simulink model "MX_Gateway"  (Simulink 9.0)
settings: Solver=FixedStepDiscrete  StartTime=0.0  StopTime=100  FixedStep=0.01  RelTol=1e-3
values from neighbouring files (symbols this model uses):
  ACTIVE = Bobcat.Parameter; CreateDefine( ACTIVE                         ,1                          ,'Define' ,'defines.h' )    [defines.m]
  ACTIVE_MODE_ONE = Bobcat.Parameter; CreateDefine( ACTIVE_MODE_ONE                ,19                         ,'Define' ,'defines.h' )    [defines.m]
  AUTHENTICATED = Bobcat.Parameter; CreateDefine( AUTHENTICATED                  ,1                          ,'Define' ,'defines.h' )    [defines.m]
  AUX_EMEA_CONTROLS = Bobcat.Parameter; CreateDefine( AUX_EMEA_CONTROLS  ,1   ,'Define' ,'defines.h' )    [enums.m]
  AUX_FLOW_HIGH = Bobcat.Parameter; CreateDefine( AUX_FLOW_HIGH           ,4  ,'Define' ,'defines.h' )    [enums.m]
  AUX_FLOW_INACTIVE = Bobcat.Parameter; CreateDefine( AUX_FLOW_INACTIVE       ,0  ,'Define' ,'defines.h' )    [enums.m]
  AUX_FLOW_LOW = Bobcat.Parameter; CreateDefine( AUX_FLOW_LOW            ,1  ,'Define' ,'defines.h' )    [enums.m]
  AUX_FLOW_MEDIUM = Bobcat.Parameter; CreateDefine( AUX_FLOW_MEDIUM         ,2  ,'Define' ,'defines.h' )    [enums.m]
  AUX_FLOW_STANDARD = Bobcat.Parameter; CreateDefine( AUX_FLOW_STANDARD       ,3  ,'Define' ,'defines.h' )    [enums.m]
  AUX_NON_FUNCTIONAL = Bobcat.Parameter; CreateDefine( AUX_NON_FUNCTIONAL ,255 ,'Define' ,'defines.h' )    [enums.m]
  BASE_ACTIVE = Bobcat.Parameter; CreateDefine( BASE_ACTIVE                    ,253                        ,'Define' ,'defines.h' )    [defines.m]
  BASE_DETENT = Bobcat.Parameter; CreateDefine( BASE_DETENT                    ,1                          ,'Define' ,'defines.h' )    [defines.m]
  BEEP_NONE = Bobcat.Parameter; CreateDefine( BEEP_NONE                      ,255                        ,'Define' ,'defines.h' )    [defines.m]
  CD_AIR_FILTER_DEBOUNCE_NORMAL = Bobcat.Parameter; CreateAliasCD( CD_AIR_FILTER_DEBOUNCE_NORMAL                        ,uint16(500)                ,'ConstantDataPointer' ,'CONSTANT_DATA' ,'AIR_FILTER_DEBOUNCE_NORMAL')    [cdata.m]    (also in hc12_cdata.m; m4_cdata.m)
  CD_AIR_FILTER_DEBOUNCE_NOT_CONNECTED = Bobcat.Parameter; CreateAliasCD( CD_AIR_FILTER_DEBOUNCE_NOT_CONNECTED                 ,uint16(3000)               ,'ConstantDataPointer' ,'CONSTANT_DATA' ,'AIR_FILTER_DEBOUNCE_NOT_CONNECTED')    [cdata.m]    (also in hc12_cdata.m; m4_cdata.m)
  CD_AIR_FILTER_DEBOUNCE_PLUGGED = Bobcat.Parameter; CreateAliasCD( CD_AIR_FILTER_DEBOUNCE_PLUGGED                       ,uint16(3000)               ,'ConstantDataPointer' ,'CONSTANT_DATA' ,'AIR_FILTER_DEBOUNCE_PLUGGED')    [cdata.m]    (also in hc12_cdata.m; m4_cdata.m)
  CD_AIR_FILTER_FUNCTION = Bobcat.Parameter; CreateAliasCD( CD_AIR_FILTER_FUNCTION                               ,uint8(255)                 ,'ConstantDataPointer' ,'CONSTANT_DATA' ,'AIR_FILTER_FUNCTION')    [cdata.m]    (also in hc12_cdata.m; m4_cdata.m)
  CD_AIR_FILTER_PLUGGED_WEIGHT = Bobcat.Parameter; CreateAliasCD( CD_AIR_FILTER_PLUGGED_WEIGHT                         ,uint8(2)                   ,'ConstantDataPointer' ,'CONSTANT_DATA' ,'AIR_FILTER_PLUGGED_WEIGHT')    [cdata.m]    (also in hc12_cdata.m; m4_cdata.m)
  CD_AIR_FILTER_UNPLUGGED_WEIGHT = Bobcat.Parameter; CreateAliasCD( CD_AIR_FILTER_UNPLUGGED_WEIGHT                       ,uint8(1)                   ,'ConstantDataPointer' ,'CONSTANT_DATA' ,'AIR_FILTER_UNPLUGGED_WEIGHT')    [cdata.m]    (also in hc12_cdata.m; m4_cdata.m)
  CD_AUTOIDLE_ARMBAR_DELAY = Bobcat.Parameter; CreateAliasCD( CD_AUTOIDLE_ARMBAR_DELAY                             ,uint8(2)                   ,'ConstantDataPointer' ,'CONSTANT_DATA' ,'AUTOIDLE_ARMBAR_DELAY')    [cdata.m]    (also in hc12_cdata.m; m4_cdata.m)
  CD_AUTOIDLE_FUNCTION = Bobcat.Parameter; CreateAliasCD( CD_AUTOIDLE_FUNCTION                                 ,uint8(255)                 ,'ConstantDataPointer' ,'CONSTANT_DATA' ,'AUTOIDLE_FUNCTION')    [cdata.m]    (also in hc12_cdata.m; m4_cdata.m)
  CD_AUTOIDLE_IDLE_DELAY = Bobcat.Parameter; CreateAliasCD( CD_AUTOIDLE_IDLE_DELAY                               ,uint8(40)                  ,'ConstantDataPointer' ,'CONSTANT_DATA' ,'AUTOIDLE_IDLE_DELAY')    [cdata.m]    (also in hc12_cdata.m; m4_cdata.m)
  CD_AUTOIDLE_LOW_IDLE_START = Bobcat.Parameter; CreateAliasCD( CD_AUTOIDLE_LOW_IDLE_START                           ,uint8(255)                 ,'ConstantDataPointer' ,'CONSTANT_DATA' ,'AUTOIDLE_LOW_IDLE_START')    [cdata.m]    (also in hc12_cdata.m; m4_cdata.m)
  CD_AUTOIDLE_PRESSURE_OFF = Bobcat.Parameter; CreateAliasCD( CD_AUTOIDLE_PRESSURE_OFF                             ,uint16(300)                ,'ConstantDataPointer' ,'CONSTANT_DATA' ,'AUTOIDLE_PRESSURE_OFF')    [cdata.m]    (also in hc12_cdata.m; m4_cdata.m)
  CD_AUTOIDLE_PRESSURE_ON = Bobcat.Parameter; CreateAliasCD( CD_AUTOIDLE_PRESSURE_ON                              ,uint16(270)                ,'ConstantDataPointer' ,'CONSTANT_DATA' ,'AUTOIDLE_PRESSURE_ON')    [cdata.m]    (also in hc12_cdata.m; m4_cdata.m)
  CD_AUTOSHIFT_FUNCTION = Bobcat.Parameter; CreateAliasCD( CD_AUTOSHIFT_FUNCTION                                ,uint8(255)                 ,'ConstantDataPointer' ,'CONSTANT_DATA' ,'AUTOSHIFT_FUNCTION')    [cdata.m]    (also in hc12_cdata.m; m4_cdata.m)
  CD_AUTOSHIFT_PHIGH1 = Bobcat.Parameter; CreateAliasCD( CD_AUTOSHIFT_PHIGH1                                  ,uint16(205)                ,'ConstantDataPointer' ,'CONSTANT_DATA' ,'AUTOSHIFT_PHIGH1')    [cdata.m]    (also in hc12_cdata.m; m4_cdata.m)
  CD_AUTOSHIFT_PHIGH2 = Bobcat.Parameter; CreateAliasCD( CD_AUTOSHIFT_PHIGH2                                  ,uint16(193)                ,'ConstantDataPointer' ,'CONSTANT_DATA' ,'AUTOSHIFT_PHIGH2')    [cdata.m]    (also in hc12_cdata.m; m4_cdata.m)
  CD_AUTOSHIFT_PHIGH_TEMP1 = Bobcat.Parameter; CreateAliasCD( CD_AUTOSHIFT_PHIGH_TEMP1                             ,int16(70)                  ,'ConstantDataPointer' ,'CONSTANT_DATA' ,'AUTOSHIFT_PHIGH_TEMP1')    [cdata.m]    (also in hc12_cdata.m; m4_cdata.m)
  CD_AUTOSHIFT_PHIGH_TEMP2 = Bobcat.Parameter; CreateAliasCD( CD_AUTOSHIFT_PHIGH_TEMP2                             ,int16(160)                 ,'ConstantDataPointer' ,'CONSTANT_DATA' ,'AUTOSHIFT_PHIGH_TEMP2')    [cdata.m]    (also in hc12_cdata.m; m4_cdata.m)
  CD_AUTOSHIFT_PLOW1 = Bobcat.Parameter; CreateAliasCD( CD_AUTOSHIFT_PLOW1                                   ,uint16(130)                ,'ConstantDataPointer' ,'CONSTANT_DATA' ,'AUTOSHIFT_PLOW1')    [cdata.m]    (also in hc12_cdata.m; m4_cdata.m)
  CD_AUTOSHIFT_PLOW2 = Bobcat.Parameter; CreateAliasCD( CD_AUTOSHIFT_PLOW2                                   ,uint16(100)                ,'ConstantDataPointer' ,'CONSTANT_DATA' ,'AUTOSHIFT_PLOW2')    [cdata.m]    (also in hc12_cdata.m; m4_cdata.m)
  CD_AUTOSHIFT_PLOW_TEMP1 = Bobcat.Parameter; CreateAliasCD( CD_AUTOSHIFT_PLOW_TEMP1                              ,int16(20)                  ,'ConstantDataPointer' ,'CONSTANT_DATA' ,'AUTOSHIFT_PLOW_TEMP1')    [cdata.m]    (also in hc12_cdata.m; m4_cdata.m)
  CD_AUTOSHIFT_PLOW_TEMP2 = Bobcat.Parameter; CreateAliasCD( CD_AUTOSHIFT_PLOW_TEMP2                              ,int16(80)                  ,'ConstantDataPointer' ,'CONSTANT_DATA' ,'AUTOSHIFT_PLOW_TEMP2')    [cdata.m]    (also in hc12_cdata.m; m4_cdata.m)
  CD_AUTOSHIFT_TRAVEL_DELAY = Bobcat.Parameter; CreateAliasCD( CD_AUTOSHIFT_TRAVEL_DELAY                            ,uint8(32)                  ,'ConstantDataPointer' ,'CONSTANT_DATA' ,'AUTOSHIFT_TRAVEL_DELAY')    [cdata.m]    (also in hc12_cdata.m; m4_cdata.m)
  CD_AUX2_CRACKPOINT_CURRENT = Bobcat.Parameter; CreateAliasCD( CD_AUX2_CRACKPOINT_CURRENT                           ,fi(0,0,16,12,'hex','0c28') ,'ConstantDataPointer' ,'CONSTANT_DATA' ,'AUX2_CRACKPOINT_CURRENT')    [cdata.m]    (also in hc12_cdata.m; m4_cdata.m)
  CD_AUX2_FLOW_LOW_FUNCTION = Bobcat.Parameter; CreateAliasCD( CD_AUX2_FLOW_LOW_FUNCTION                            ,uint8(255)                 ,'ConstantDataPointer' ,'CONSTANT_DATA' ,'AUX2_FLOW_LOW_FUNCTION')    [cdata.m]    (also in hc12_cdata.m; m4_cdata.m)
  CD_AUX2_FLOW_MED_FUNCTION = Bobcat.Parameter; CreateAliasCD( CD_AUX2_FLOW_MED_FUNCTION                            ,uint8(255)                 ,'ConstantDataPointer' ,'CONSTANT_DATA' ,'AUX2_FLOW_MED_FUNCTION')    [cdata.m]    (also in hc12_cdata.m; m4_cdata.m)
  CD_AUX2_HI_FLOW_PERCENT = Bobcat.Parameter; CreateAliasCD( CD_AUX2_HI_FLOW_PERCENT                              ,fi(0,0,16,16,'hex','ffff') ,'ConstantDataPointer' ,'CONSTANT_DATA' ,'AUX2_HI_FLOW_PERCENT')    [cdata.m]    (also in hc12_cdata.m; m4_cdata.m)
  CD_AUX2_LOW_FLOW_PERCENT = Bobcat.Parameter; CreateAliasCD( CD_AUX2_LOW_FLOW_PERCENT                             ,fi(0,0,16,16,'hex','7333') ,'ConstantDataPointer' ,'CONSTANT_DATA' ,'AUX2_LOW_FLOW_PERCENT')    [cdata.m]    (also in hc12_cdata.m; m4_cdata.m)
  CD_AUX2_MAX_FLOW_CURRENT = Bobcat.Parameter; CreateAliasCD( CD_AUX2_MAX_FLOW_CURRENT                             ,fi(0,0,16,12,'hex','14cc') ,'ConstantDataPointer' ,'CONSTANT_DATA' ,'AUX2_MAX_FLOW_CURRENT')    [cdata.m]    (also in hc12_cdata.m; m4_cdata.m)
  CD_AUX2_MED_FLOW_PERCENT = Bobcat.Parameter; CreateAliasCD( CD_AUX2_MED_FLOW_PERCENT                             ,fi(0,0,16,16,'hex','9999') ,'ConstantDataPointer' ,'CONSTANT_DATA' ,'AUX2_MED_FLOW_PERCENT')    [cdata.m]    (also in hc12_cdata.m; m4_cdata.m)
  CD_AUX_CRACKPOINT_CURRENT = Bobcat.Parameter; CreateAliasCD( CD_AUX_CRACKPOINT_CURRENT                            ,fi(0,0,16,12,'hex','0000') ,'ConstantDataPointer' ,'CONSTANT_DATA' ,'AUX_CRACKPOINT_CURRENT')    [cdata.m]    (also in hc12_cdata.m; m4_cdata.m)
  CD_AUX_DETENT_FLASH_TIME = Bobcat.Parameter; CreateAliasCD( CD_AUX_DETENT_FLASH_TIME                             ,uint16(72)                 ,'ConstantDataPointer' ,'CONSTANT_DATA' ,'AUX_DETENT_FLASH_TIME')    [cdata.m]    (also in hc12_cdata.m; m4_cdata.m)
  CD_AUX_DETENT_HOLD_TIME = Bobcat.Parameter; CreateAliasCD( CD_AUX_DETENT_HOLD_TIME                              ,uint16(100)                ,'ConstantDataPointer' ,'CONSTANT_DATA' ,'AUX_DETENT_HOLD_TIME')    [cdata.m]    (also in hc12_cdata.m; m4_cdata.m)
  CD_AUX_FULL_ON_AUX1 = Bobcat.Parameter; CreateAliasCD( CD_AUX_FULL_ON_AUX1                                  ,fi(0,0,16,12,'hex','10cd') ,'ConstantDataPointer' ,'CONSTANT_DATA' ,'AUX_FULL_ON_AUX1')    [cdata.m]    (also in hc12_cdata.m; m4_cdata.m)
  CD_AUX_FULL_ON_AUX2 = Bobcat.Parameter; CreateAliasCD( CD_AUX_FULL_ON_AUX2                                  ,fi(0,0,16,12,'hex','139d') ,'ConstantDataPointer' ,'CONSTANT_DATA' ,'AUX_FULL_ON_AUX2')    [cdata.m]    (also in hc12_cdata.m; m4_cdata.m)
  CD_AUX_FULL_ON_AUX3 = Bobcat.Parameter; CreateAliasCD( CD_AUX_FULL_ON_AUX3                                  ,fi(0,0,16,12,'hex','17d7') ,'ConstantDataPointer' ,'CONSTANT_DATA' ,'AUX_FULL_ON_AUX3')    [cdata.m]    (also in hc12_cdata.m; m4_cdata.m)
  CD_AUX_FUNCTION = Bobcat.Parameter; CreateAliasCD( CD_AUX_FUNCTION                                      ,uint8(0)                   ,'ConstantDataPointer' ,'CONSTANT_DATA' ,'AUX_FUNCTION')    [cdata.m]    (also in hc12_cdata.m; m4_cdata.m)
  CD_AUX_RATE_LIMIT_CRACKPOINT = Bobcat.Parameter; CreateAliasCD( CD_AUX_RATE_LIMIT_CRACKPOINT                         ,fi(0,0,16,12,'hex','0800') ,'ConstantDataPointer' ,'CONSTANT_DATA' ,'AUX_RATE_LIMIT_CRACKPOINT')    [cdata.m]    (also in hc12_cdata.m; m4_cdata.m)
  CD_AUX_RATE_LIMIT_CRACKPOINT_RATE = Bobcat.Parameter; CreateAliasCD( CD_AUX_RATE_LIMIT_CRACKPOINT_RATE                    ,fi(0,1,32,12,'int',102400) ,'ConstantDataPointer' ,'CONSTANT_DATA' ,'AUX_RATE_LIMIT_CRACKPOINT_RATE')    [cdata.m]    (also in hc12_cdata.m; m4_cdata.m)
  CD_AUX_RATE_LIMIT_FUNCTION = Bobcat.Parameter; CreateAliasCD( CD_AUX_RATE_LIMIT_FUNCTION                           ,uint8(255)                 ,'ConstantDataPointer' ,'CONSTANT_DATA' ,'AUX_RATE_LIMIT_FUNCTION')    [cdata.m]    (also in hc12_cdata.m; m4_cdata.m)
  CD_AUX_RATE_LIMIT_MAX_ENG_SPD = Bobcat.Parameter; CreateAliasCD( CD_AUX_RATE_LIMIT_MAX_ENG_SPD                        ,uint16(1600)               ,'ConstantDataPointer' ,'CONSTANT_DATA' ,'AUX_RATE_LIMIT_MAX_ENG_SPD')    [cdata.m]    (also in hc12_cdata.m; m4_cdata.m)
  CD_AUX_RATE_LIMIT_MIN_ENG_SPD = Bobcat.Parameter; CreateAliasCD( CD_AUX_RATE_LIMIT_MIN_ENG_SPD                        ,uint16(100)                ,'ConstantDataPointer' ,'CONSTANT_DATA' ,'AUX_RATE_LIMIT_MIN_ENG_SPD')    [cdata.m]    (also in hc12_cdata.m; m4_cdata.m)
  CD_AUX_RATE_LIMIT_NORMAL_RATE = Bobcat.Parameter; CreateAliasCD( CD_AUX_RATE_LIMIT_NORMAL_RATE                        ,fi(0,1,32,12,'int',2048)   ,'ConstantDataPointer' ,'CONSTANT_DATA' ,'AUX_RATE_LIMIT_NORMAL_RATE')    [cdata.m]    (also in hc12_cdata.m; m4_cdata.m)
  CD_AUXPOT_CAL_DEADBAND_HIGH = Bobcat.Parameter; CreateAliasCD( CD_AUXPOT_CAL_DEADBAND_HIGH                          ,uint16(574)                ,'ConstantDataPointer' ,'CONSTANT_DATA' ,'AUXPOT_CAL_DEADBAND_HIGH')    [cdata.m]    (also in hc12_cdata.m; m4_cdata.m)
  CD_AUXPOT_CAL_DEADBAND_LOW = Bobcat.Parameter; CreateAliasCD( CD_AUXPOT_CAL_DEADBAND_LOW                           ,uint16(424)                ,'ConstantDataPointer' ,'CONSTANT_DATA' ,'AUXPOT_CAL_DEADBAND_LOW')    [cdata.m]    (also in hc12_cdata.m; m4_cdata.m)
  CD_AUXPOT_CENTER_RATIO = Bobcat.Parameter; CreateAliasCD( CD_AUXPOT_CENTER_RATIO                               ,uint16(500)                ,'ConstantDataPointer' ,'CONSTANT_DATA' ,'AUXPOT_CENTER_RATIO')    [cdata.m]    (also in hc12_cdata.m; m4_cdata.m)
  CD_AUXPOT_DEADBAND = Bobcat.Parameter; CreateAliasCD( CD_AUXPOT_DEADBAND                                   ,uint8(38)                  ,'ConstantDataPointer' ,'CONSTANT_DATA' ,'AUXPOT_DEADBAND')    [cdata.m]    (also in hc12_cdata.m; m4_cdata.m)
  CD_AUXPOT_FUNCTION = Bobcat.Parameter; CreateAliasCD( CD_AUXPOT_FUNCTION                                   ,uint8(0)                   ,'ConstantDataPointer' ,'CONSTANT_DATA' ,'AUXPOT_FUNCTION')    [cdata.m]    (also in hc12_cdata.m; m4_cdata.m)
  CD_AUXPOT_RATIO_GAIN = Bobcat.Parameter; CreateAliasCD( CD_AUXPOT_RATIO_GAIN                                 ,uint16(1000)               ,'ConstantDataPointer' ,'CONSTANT_DATA' ,'AUXPOT_RATIO_GAIN')    [cdata.m]    (also in hc12_cdata.m; m4_cdata.m)
  CD_CAN_THROTTLE_FUNCTION = Bobcat.Parameter; CreateAliasCD( CD_CAN_THROTTLE_FUNCTION                             ,uint8(255)                 ,'ConstantDataPointer' ,'CONSTANT_DATA' ,'CAN_THROTTLE_FUNCTION')    [cdata.m]    (also in hc12_cdata.m; m4_cdata.m)
  CD_CAN_THROTTLE_REVERSE = Bobcat.Parameter; CreateAliasCD( CD_CAN_THROTTLE_REVERSE                              ,uint8(255)                 ,'ConstantDataPointer' ,'CONSTANT_DATA' ,'CAN_THROTTLE_REVERSE')    [cdata.m]    (also in hc12_cdata.m; m4_cdata.m)
  CD_CONSOLE_DEBOUNCE_ABSENT = Bobcat.Parameter; CreateAliasCD( CD_CONSOLE_DEBOUNCE_ABSENT                           ,uint16(12)                 ,'ConstantDataPointer' ,'CONSTANT_DATA' ,'CONSOLE_DEBOUNCE_ABSENT')    [cdata.m]    (also in hc12_cdata.m; m4_cdata.m)
  CD_CONSOLE_DEBOUNCE_OORH = Bobcat.Parameter; CreateAliasCD( CD_CONSOLE_DEBOUNCE_OORH                             ,uint16(12)                 ,'ConstantDataPointer' ,'CONSTANT_DATA' ,'CONSOLE_DEBOUNCE_OORH')    [cdata.m]    (also in hc12_cdata.m; m4_cdata.m)
  CD_CONSOLE_DEBOUNCE_OORL = Bobcat.Parameter; CreateAliasCD( CD_CONSOLE_DEBOUNCE_OORL                             ,uint16(12)                 ,'ConstantDataPointer' ,'CONSTANT_DATA' ,'CONSOLE_DEBOUNCE_OORL')    [cdata.m]    (also in hc12_cdata.m; m4_cdata.m)
  CD_CONSOLE_DEBOUNCE_PRESENT = Bobcat.Parameter; CreateAliasCD( CD_CONSOLE_DEBOUNCE_PRESENT                          ,uint16(12)                 ,'ConstantDataPointer' ,'CONSTANT_DATA' ,'CONSOLE_DEBOUNCE_PRESENT')    [cdata.m]    (also in hc12_cdata.m; m4_cdata.m)
  CD_CONSOLE_FUNCTION = Bobcat.Parameter; CreateAliasCD( CD_CONSOLE_FUNCTION                                  ,uint8(0)                   ,'ConstantDataPointer' ,'CONSTANT_DATA' ,'CONSOLE_FUNCTION')    [cdata.m]    (also in hc12_cdata.m; m4_cdata.m)
  CD_CONSOLE_OORH = Bobcat.Parameter; CreateAliasCD( CD_CONSOLE_OORH                                      ,uint16(740)                ,'ConstantDataPointer' ,'CONSTANT_DATA' ,'CONSOLE_OORH')    [cdata.m]    (also in hc12_cdata.m; m4_cdata.m)
  CD_CONSOLE_OORL = Bobcat.Parameter; CreateAliasCD( CD_CONSOLE_OORL                                      ,uint16(10)                 ,'ConstantDataPointer' ,'CONSTANT_DATA' ,'CONSOLE_OORL')    [cdata.m]    (also in hc12_cdata.m; m4_cdata.m)
  CD_CONSOLE_PRESENT_HIGH = Bobcat.Parameter; CreateAliasCD( CD_CONSOLE_PRESENT_HIGH                              ,uint16(515)                ,'ConstantDataPointer' ,'CONSTANT_DATA' ,'CONSOLE_PRESENT_HIGH')    [cdata.m]    (also in hc12_cdata.m; m4_cdata.m)
  CD_CONSOLE_PRESENT_LOW = Bobcat.Parameter; CreateAliasCD( CD_CONSOLE_PRESENT_LOW                               ,uint16(375)                ,'ConstantDataPointer' ,'CONSTANT_DATA' ,'CONSOLE_PRESENT_LOW')    [cdata.m]    (also in hc12_cdata.m; m4_cdata.m)
  CD_DELUXE_GEN5_5_FUNCTION = Bobcat.Parameter; CreateAliasCD( CD_DELUXE_GEN5_5_FUNCTION                            ,uint8(255)                 ,'ConstantDataPointer' ,'CONSTANT_DATA' ,'DELUXE_GEN5_5_FUNCTION')    [cdata.m]    (also in hc12_cdata.m; m4_cdata.m)
  CD_DELUXE_GEN5_FUNCTION = Bobcat.Parameter; CreateAliasCD( CD_DELUXE_GEN5_FUNCTION                              ,uint8(255)                 ,'ConstantDataPointer' ,'CONSTANT_DATA' ,'DELUXE_GEN5_FUNCTION')    [cdata.m]    (also in hc12_cdata.m; m4_cdata.m)
  CD_DERATE_ENGINE_SPEED_DEBOUNCE_HIGH = Bobcat.Parameter; CreateAliasCD( CD_DERATE_ENGINE_SPEED_DEBOUNCE_HIGH                 ,uint16(200)                ,'ConstantDataPointer' ,'CONSTANT_DATA' ,'DERATE_ENGINE_SPEED_DEBOUNCE_HIGH')    [cdata.m]    (also in hc12_cdata.m; m4_cdata.m)
  CD_DERATE_ENGINE_SPEED_DEBOUNCE_SHUTDOWN = Bobcat.Parameter; CreateAliasCD( CD_DERATE_ENGINE_SPEED_DEBOUNCE_SHUTDOWN             ,uint16(1000)               ,'ConstantDataPointer' ,'CONSTANT_DATA' ,'DERATE_ENGINE_SPEED_DEBOUNCE_SHUTDOWN')    [cdata.m]    (also in hc12_cdata.m; m4_cdata.m)
  CD_DERATE_ENGINE_SPEED_HIGH_OFFSET = Bobcat.Parameter; CreateAliasCD( CD_DERATE_ENGINE_SPEED_HIGH_OFFSET                   ,uint16(200)                ,'ConstantDataPointer' ,'CONSTANT_DATA' ,'DERATE_ENGINE_SPEED_HIGH_OFFSET')    [cdata.m]    (also in hc12_cdata.m; m4_cdata.m)
  CD_DERATE_METHOD = Bobcat.Parameter; CreateAliasCD( CD_DERATE_METHOD                                     ,uint8(0)                   ,'ConstantDataPointer' ,'CONSTANT_DATA' ,'DERATE_METHOD')    [cdata.m]    (also in hc12_cdata.m; m4_cdata.m)
  CD_DUMP_VALVE_FUNCTION = Bobcat.Parameter; CreateAliasCD( CD_DUMP_VALVE_FUNCTION                               ,uint8(255)                 ,'ConstantDataPointer' ,'CONSTANT_DATA' ,'DUMP_VALVE_FUNCTION')    [cdata.m]    (also in hc12_cdata.m; m4_cdata.m)
  CD_DUMP_VALVE_ON_CURRENT = Bobcat.Parameter; CreateAliasCD( CD_DUMP_VALVE_ON_CURRENT                             ,fi(0,0,16,12,'hex','ffff') ,'ConstantDataPointer' ,'CONSTANT_DATA' ,'DUMP_VALVE_ON_CURRENT')    [cdata.m]    (also in hc12_cdata.m; m4_cdata.m)
  CD_ECO_MODE_THROTTLE_REDUCTION = Bobcat.Parameter; CreateAliasCD( CD_ECO_MODE_THROTTLE_REDUCTION                       ,uint16(850)                ,'ConstantDataPointer' ,'CONSTANT_DATA' ,'ECO_MODE_THROTTLE_REDUCTION')    [cdata.m]    (also in hc12_cdata.m; m4_cdata.m)
  CD_ECT_FILTER_GAIN = Bobcat.Parameter; CreateAliasCD( CD_ECT_FILTER_GAIN                                   ,uint8(6)                   ,'ConstantDataPointer' ,'CONSTANT_DATA' ,'ECT_FILTER_GAIN')    [cdata.m]    (also in hc12_cdata.m; m4_cdata.m)
  CD_ECU_FUNCTION = Bobcat.Parameter; CreateAliasCD( CD_ECU_FUNCTION                                      ,uint8(255)                 ,'ConstantDataPointer' ,'CONSTANT_DATA' ,'ECU_FUNCTION')    [cdata.m]    (also in hc12_cdata.m; m4_cdata.m)
  CD_ECU_HOLD_DEBOUNCE_ERROR_ON = Bobcat.Parameter; CreateAliasCD( CD_ECU_HOLD_DEBOUNCE_ERROR_ON                        ,uint16(20)                 ,'ConstantDataPointer' ,'CONSTANT_DATA' ,'ECU_HOLD_DEBOUNCE_ERROR_ON')    [cdata.m]    (also in hc12_cdata.m; m4_cdata.m)
  CD_EMEA_CONTROLS_HOLD_TIME = Bobcat.Parameter; CreateAliasCD( CD_EMEA_CONTROLS_HOLD_TIME                           ,uint16(30)                 ,'ConstantDataPointer' ,'CONSTANT_DATA' ,'EMEA_CONTROLS_HOLD_TIME')    [cdata.m]    (also in hc12_cdata.m; m4_cdata.m)
  CD_EMEA_CONTROLS_OPTION_A = Bobcat.Parameter; CreateAliasCD( CD_EMEA_CONTROLS_OPTION_A                            ,uint8(255)                 ,'ConstantDataPointer' ,'CONSTANT_DATA' ,'EMEA_CONTROLS_OPTION_A')    [cdata.m]    (also in hc12_cdata.m; m4_cdata.m)
  CD_EMEA_CONTROLS_OPTION_C = Bobcat.Parameter; CreateAliasCD( CD_EMEA_CONTROLS_OPTION_C                            ,uint8(255)                 ,'ConstantDataPointer' ,'CONSTANT_DATA' ,'EMEA_CONTROLS_OPTION_C')    [cdata.m]    (also in hc12_cdata.m; m4_cdata.m)
  CD_EMEA_CONTROLS_REVERSE = Bobcat.Parameter; CreateAliasCD( CD_EMEA_CONTROLS_REVERSE                             ,uint8(255)                 ,'ConstantDataPointer' ,'CONSTANT_DATA' ,'EMEA_CONTROLS_REVERSE')    [cdata.m]    (also in hc12_cdata.m; m4_cdata.m)
  CD_EMEA_ELECTRIC_VERSION = Bobcat.Parameter; CreateAliasCD( CD_EMEA_ELECTRIC_VERSION                             ,uint8(0)                   ,'ConstantDataPointer' ,'CONSTANT_DATA' ,'EMEA_ELECTRIC_VERSION')    [cdata.m]    (also in hc12_cdata.m; m4_cdata.m)
  CD_EMEA_POT_CAL_DEADBAND_HIGH = Bobcat.Parameter; CreateAliasCD( CD_EMEA_POT_CAL_DEADBAND_HIGH                        ,uint16(574)                ,'ConstantDataPointer' ,'CONSTANT_DATA' ,'EMEA_POT_CAL_DEADBAND_HIGH')    [cdata.m]    (also in hc12_cdata.m; m4_cdata.m)
  CD_EMEA_POT_CAL_DEADBAND_LOW = Bobcat.Parameter; CreateAliasCD( CD_EMEA_POT_CAL_DEADBAND_LOW                         ,uint16(424)                ,'ConstantDataPointer' ,'CONSTANT_DATA' ,'EMEA_POT_CAL_DEADBAND_LOW')    [cdata.m]    (also in hc12_cdata.m; m4_cdata.m)
  CD_ENGINE_RPM_FACTOR = Bobcat.Parameter; CreateAliasCD( CD_ENGINE_RPM_FACTOR                                 ,uint8(35)                  ,'ConstantDataPointer' ,'CONSTANT_DATA' ,'ENGINE_RPM_FACTOR')    [cdata.m]    (also in hc12_cdata.m; m4_cdata.m)
  CD_ENGINE_SPEED_FILTER_FAST_VALUE = Bobcat.Parameter; CreateAliasCD( CD_ENGINE_SPEED_FILTER_FAST_VALUE                    ,uint8(1)                   ,'ConstantDataPointer' ,'CONSTANT_DATA' ,'ENGINE_SPEED_FILTER_FAST_VALUE')    [cdata.m]    (also in hc12_cdata.m; m4_cdata.m)
  CD_ENGINE_SPEED_FILTER_SLOW_VALUE = Bobcat.Parameter; CreateAliasCD( CD_ENGINE_SPEED_FILTER_SLOW_VALUE                    ,uint8(4)                   ,'ConstantDataPointer' ,'CONSTANT_DATA' ,'ENGINE_SPEED_FILTER_SLOW_VALUE')    [cdata.m]    (also in hc12_cdata.m; m4_cdata.m)
  CD_ENGINE_SPEED_RUNNING = Bobcat.Parameter; CreateAliasCD( CD_ENGINE_SPEED_RUNNING                              ,uint16(800)                ,'ConstantDataPointer' ,'CONSTANT_DATA' ,'ENGINE_SPEED_RUNNING')    [cdata.m]    (also in hc12_cdata.m; m4_cdata.m)
  CD_ENGINE_START_DELAY_DEBOUNCE_RPM_NO_SIGNAL = Bobcat.Parameter; CreateAliasCD( CD_ENGINE_START_DELAY_DEBOUNCE_RPM_NO_SIGNAL         ,uint16(0)                  ,'ConstantDataPointer' ,'CONSTANT_DATA' ,'ENGINE_START_DELAY_DEBOUNCE_RPM_NO_SIGNAL')    [cdata.m]    (also in hc12_cdata.m; m4_cdata.m)
  CD_ENGINE_START_DELAY_OFF_TIME = Bobcat.Parameter; CreateAliasCD( CD_ENGINE_START_DELAY_OFF_TIME                       ,uint16(0)                  ,'ConstantDataPointer' ,'CONSTANT_DATA' ,'ENGINE_START_DELAY_OFF_TIME')    [cdata.m]    (also in hc12_cdata.m; m4_cdata.m)
  CD_ENGINE_START_DELAY_RPM_NO_SIGNAL = Bobcat.Parameter; CreateAliasCD( CD_ENGINE_START_DELAY_RPM_NO_SIGNAL                  ,uint16(0)                  ,'ConstantDataPointer' ,'CONSTANT_DATA' ,'ENGINE_START_DELAY_RPM_NO_SIGNAL')    [cdata.m]    (also in hc12_cdata.m; m4_cdata.m)
  CD_EOP_DEBOUNCE_EXTREMELY_LOW = Bobcat.Parameter; CreateAliasCD( CD_EOP_DEBOUNCE_EXTREMELY_LOW                        ,uint16(50)                 ,'ConstantDataPointer' ,'CONSTANT_DATA' ,'EOP_DEBOUNCE_EXTREMELY_LOW')    [cdata.m]    (also in hc12_cdata.m; m4_cdata.m)
  CD_EOP_DEBOUNCE_NORMAL = Bobcat.Parameter; CreateAliasCD( CD_EOP_DEBOUNCE_NORMAL                               ,uint16(50)                 ,'ConstantDataPointer' ,'CONSTANT_DATA' ,'EOP_DEBOUNCE_NORMAL')    [cdata.m]    (also in hc12_cdata.m; m4_cdata.m)
  CD_EOP_DEBOUNCE_SHUTDOWN = Bobcat.Parameter; CreateAliasCD( CD_EOP_DEBOUNCE_SHUTDOWN                             ,uint16(100)                ,'ConstantDataPointer' ,'CONSTANT_DATA' ,'EOP_DEBOUNCE_SHUTDOWN')    [cdata.m]    (also in hc12_cdata.m; m4_cdata.m)
  CD_EOP_FILTER_VALUE = Bobcat.Parameter; CreateAliasCD( CD_EOP_FILTER_VALUE                                  ,uint8(3)                   ,'ConstantDataPointer' ,'CONSTANT_DATA' ,'EOP_FILTER_VALUE')    [cdata.m]    (also in hc12_cdata.m; m4_cdata.m)
  CD_EOP_FUNCTION = Bobcat.Parameter; CreateAliasCD( CD_EOP_FUNCTION                                      ,uint8(1)                   ,'ConstantDataPointer' ,'CONSTANT_DATA' ,'EOP_FUNCTION')    [cdata.m]    (also in hc12_cdata.m; m4_cdata.m)
  CD_EOP_SWITCH_GAUGE_VALUE = Bobcat.Parameter; CreateAliasCD( CD_EOP_SWITCH_GAUGE_VALUE                            ,uint16(125)                ,'ConstantDataPointer' ,'CONSTANT_DATA' ,'EOP_SWITCH_GAUGE_VALUE')    [cdata.m]    (also in hc12_cdata.m; m4_cdata.m)
  CD_EOP_SWITCH_LOW = Bobcat.Parameter; CreateAliasCD( CD_EOP_SWITCH_LOW                                    ,uint16(50)                 ,'ConstantDataPointer' ,'CONSTANT_DATA' ,'EOP_SWITCH_LOW')    [cdata.m]    (also in hc12_cdata.m; m4_cdata.m)
  CD_EOP_SWITCH_PRESSURE_VALUE = Bobcat.Parameter; CreateAliasCD( CD_EOP_SWITCH_PRESSURE_VALUE                         ,uint16(40)                 ,'ConstantDataPointer' ,'CONSTANT_DATA' ,'EOP_SWITCH_PRESSURE_VALUE')    [cdata.m]    (also in hc12_cdata.m; m4_cdata.m)
  CD_EOT_DEBOUNCE_EXTREMELY_LOW = Bobcat.Parameter; CreateAliasCD( CD_EOT_DEBOUNCE_EXTREMELY_LOW                        ,uint16(2)                  ,'ConstantDataPointer' ,'CONSTANT_DATA' ,'EOT_DEBOUNCE_EXTREMELY_LOW')    [cdata.m]    (also in hc12_cdata.m; m4_cdata.m)
  CD_EOT_DEBOUNCE_LOW = Bobcat.Parameter; CreateAliasCD( CD_EOT_DEBOUNCE_LOW                                  ,uint16(2)                  ,'ConstantDataPointer' ,'CONSTANT_DATA' ,'EOT_DEBOUNCE_LOW')    [cdata.m]    (also in hc12_cdata.m; m4_cdata.m)
  CD_EOT_DEBOUNCE_NORMAL = Bobcat.Parameter; CreateAliasCD( CD_EOT_DEBOUNCE_NORMAL                               ,uint16(2)                  ,'ConstantDataPointer' ,'CONSTANT_DATA' ,'EOT_DEBOUNCE_NORMAL')    [cdata.m]    (also in hc12_cdata.m; m4_cdata.m)
  CD_EOT_EXTREMELY_LOW = Bobcat.Parameter; CreateAliasCD( CD_EOT_EXTREMELY_LOW                                 ,uint16(81)                 ,'ConstantDataPointer' ,'CONSTANT_DATA' ,'EOT_EXTREMELY_LOW')    [cdata.m]    (also in hc12_cdata.m; m4_cdata.m)
  CD_EOT_FUNCTION = Bobcat.Parameter; CreateAliasCD( CD_EOT_FUNCTION                                      ,uint8(255)                 ,'ConstantDataPointer' ,'CONSTANT_DATA' ,'EOT_FUNCTION')    [cdata.m]    (also in hc12_cdata.m; m4_cdata.m)
  CD_EOT_LOW = Bobcat.Parameter; CreateAliasCD( CD_EOT_LOW                                           ,uint16(90)                 ,'ConstantDataPointer' ,'CONSTANT_DATA' ,'EOT_LOW')    [cdata.m]    (also in hc12_cdata.m; m4_cdata.m)
  CD_EOT_TEMPERATURE_HYSTERESIS = Bobcat.Parameter; CreateAliasCD( CD_EOT_TEMPERATURE_HYSTERESIS                        ,uint16(2)                  ,'ConstantDataPointer' ,'CONSTANT_DATA' ,'EOT_TEMPERATURE_HYSTERESIS')    [cdata.m]    (also in hc12_cdata.m; m4_cdata.m)
  CD_FAST_TRAVEL_FUNCTION = Bobcat.Parameter; CreateAliasCD( CD_FAST_TRAVEL_FUNCTION                              ,uint8(255)                 ,'ConstantDataPointer' ,'CONSTANT_DATA' ,'FAST_TRAVEL_FUNCTION')    [cdata.m]    (also in hc12_cdata.m; m4_cdata.m)
  CD_FAST_TRAVEL_THROTTLE_DELAY = Bobcat.Parameter; CreateAliasCD( CD_FAST_TRAVEL_THROTTLE_DELAY                        ,uint16(10)                 ,'ConstantDataPointer' ,'CONSTANT_DATA' ,'FAST_TRAVEL_THROTTLE_DELAY')    [cdata.m]    (also in hc12_cdata.m; m4_cdata.m)
  CD_FAST_TRAVEL_THROTTLE_LIMIT = Bobcat.Parameter; CreateAliasCD( CD_FAST_TRAVEL_THROTTLE_LIMIT                        ,uint16(1000)               ,'ConstantDataPointer' ,'CONSTANT_DATA' ,'FAST_TRAVEL_THROTTLE_LIMIT')    [cdata.m]    (also in hc12_cdata.m; m4_cdata.m)
  CD_FLYWHEEL_TEETH = Bobcat.Parameter; CreateAliasCD( CD_FLYWHEEL_TEETH                                    ,uint8(83)                  ,'ConstantDataPointer' ,'CONSTANT_DATA' ,'FLYWHEEL_TEETH')    [cdata.m]    (also in hc12_cdata.m; m4_cdata.m)
  CD_FUEL_LEVEL_FILTER_FAST_VALUE = Bobcat.Parameter; CreateAliasCD( CD_FUEL_LEVEL_FILTER_FAST_VALUE                      ,uint8(5)                   ,'ConstantDataPointer' ,'CONSTANT_DATA' ,'FUEL_LEVEL_FILTER_FAST_VALUE')    [cdata.m]    (also in hc12_cdata.m; m4_cdata.m)
  CD_FUEL_LEVEL_FILTER_SLOW_VALUE = Bobcat.Parameter; CreateAliasCD( CD_FUEL_LEVEL_FILTER_SLOW_VALUE                      ,uint8(10)                  ,'ConstantDataPointer' ,'CONSTANT_DATA' ,'FUEL_LEVEL_FILTER_SLOW_VALUE')    [cdata.m]    (also in hc12_cdata.m; m4_cdata.m)
  CD_FUEL_PRIMARY_FEEDBACK_FUNCTION = Bobcat.Parameter; CreateAliasCD( CD_FUEL_PRIMARY_FEEDBACK_FUNCTION                    ,uint8(0)                   ,'ConstantDataPointer' ,'CONSTANT_DATA' ,'FUEL_PRIMARY_FEEDBACK_FUNCTION')    [cdata.m]    (also in hc12_cdata.m; m4_cdata.m)
  ... (+246 more)
undefined symbols (not defined in the file or in its folder): sigbuilder_block
undefined symbols (not defined in the file): CD_FUEL_RESOLUTION_TIMER
libraries: simulink x186, MX_Library x107
(part 43 of 48)

== stateflow chart MX_Gtwy_Control/PanelInterface/Keyless_Indicators/Keyless_Ind ==
  settings: executeAtInitialization=1
  state LOCK [parallel]
    settings: executionOrder=1
    state ACTIVE
      en:ind_Lock = ACTIVE;
    state INACTIVE
      en:ind_Lock = INACTIVE;
    state FAIL
      state ACTIVE
        en:ind_Lock = ACTIVE;
      state INACTIVE
        en:ind_Lock = INACTIVE;
      (default) -> LOCK.FAIL.ACTIVE
      LOCK.FAIL.INACTIVE -> LOCK.FAIL.ACTIVE : after(CD_ICON_FLASH_RATE, tick)
      LOCK.FAIL.ACTIVE -> LOCK.FAIL.INACTIVE : after(CD_ICON_FLASH_RATE,tick)
    (default) -> LOCK.INACTIVE
    LOCK.INACTIVE -> LOCK.ACTIVE [order 1] : [(ee_PasswordLock == ACTIVE)]
    LOCK.ACTIVE -> LOCK.INACTIVE [order 2] : [(ee_PasswordLock == INACTIVE)]
    LOCK.FAIL -> LOCK.ACTIVE [order 1] : [(state_KSPassword != FAIL) &&...
        |  (state_KSPassword != ENTER_PWD) &&...
        |  (ee_PasswordLock == ACTIVE) &&...
        |  (state_KSLock != KS_LOCK_MACHINE) &&...
        |  (state_KSLock != KS_CHANGE)]
    LOCK.ACTIVE -> LOCK.FAIL [order 1] : [(state_KSPassword == FAIL) ||...
        |  ((state_KSPassword == ENTER_PWD) &&...
        |  (state_KSLock != KS_UNLOCK_MACHINE)) ||...
        |  (state_KSLock == KS_CHANGE)]
    LOCK.INACTIVE -> LOCK.FAIL [order 2] : [(state_KSPassword == FAIL)...
        | || (state_KSLock == KS_LOCK_MACHINE)...
        | || (state_KSLock == KS_CHANGE)]
    LOCK.FAIL -> LOCK.INACTIVE [order 2] : [(state_KSPassword != FAIL) &&...
        |  (state_KSPassword != ENTER_PWD) &&...
        |  (ee_PasswordLock == INACTIVE) &&...
        |  (state_KSLock != KS_LOCK_MACHINE) &&...
        |  (state_KSLock != KS_CHANGE)]
  state UNLOCK [parallel]
    settings: executionOrder=2
    state INACTIVE
      en:ind_Unlock = INACTIVE;
    state ACTIVE
      en:ind_Unlock = ACTIVE;
    state FAIL
      state INACTIVE
        en:ind_Unlock = INACTIVE;
      state ACTIVE
        en:ind_Unlock = ACTIVE;
      (default) -> UNLOCK.FAIL.ACTIVE
      UNLOCK.FAIL.ACTIVE -> UNLOCK.FAIL.INACTIVE : after(CD_ICON_FLASH_RATE,tick)
      UNLOCK.FAIL.INACTIVE -> UNLOCK.FAIL.ACTIVE : after(CD_ICON_FLASH_RATE, tick)
    (default) -> UNLOCK.INACTIVE
    UNLOCK.INACTIVE -> UNLOCK.ACTIVE [order 1] : [(ee_PasswordLock == INACTIVE)]
    UNLOCK.ACTIVE -> UNLOCK.INACTIVE [order 2] : [(ee_PasswordLock == ACTIVE)]
    UNLOCK.FAIL -> UNLOCK.INACTIVE [order 1] : [(state_KSPassword != FAIL) &&...
        |  (ee_PasswordLock == ACTIVE) &&...
        |  (state_KSLock != KS_UNLOCK_MACHINE) &&...
        |  (state_KSLock != KS_CHANGE)]
    UNLOCK.INACTIVE -> UNLOCK.FAIL [order 2] : [(state_KSPassword == FAIL) ||...
        |  (state_KSLock == KS_UNLOCK_MACHINE) ||...
        |  (state_KSLock == KS_CHANGE)]
    UNLOCK.FAIL -> UNLOCK.ACTIVE [order 2] : [(state_KSPassword != FAIL) &&...
        |  (ee_PasswordLock == INACTIVE) &&...
        |  (state_KSLock != KS_UNLOCK_MACHINE) &&...
        |  (state_KSLock != KS_CHANGE)]
    UNLOCK.ACTIVE -> UNLOCK.FAIL [order 1] : [(state_KSPassword == FAIL) ||...
        |  (state_KSLock == KS_CHANGE)]
  data: ind_Lock output boolean; ACTIVE parameter uint8; INACTIVE parameter uint8; ind_Unlock output boolean; FAIL parameter uint8; state_KSPassword input uint8; CD_ICON_FLASH_RATE parameter uint16; state_KSLock input uint8; KS_LOCK_MACHINE parameter uint8; KS_CHANGE parameter uint8; KS_UNLOCK_MACHINE parameter uint8; ee_PasswordLock data_store_memory double; ENTER_PWD parameter uint8

== stateflow chart MX_Gtwy_Control/Inputs/Eco_Mode/Eco_Mode ==
  state Normal
    state On
      en:
      state_EcoMode = ACTIVE;
      ee_EcoMode = ACTIVE;
      matlab_UpdateEEVar();
    state Init
      en:
      state_EcoMode = INACTIVE;
    state Off
      en:
      state_EcoMode = INACTIVE;
      ee_EcoMode = INACTIVE;
      matlab_UpdateEEVar();
    (default) -> Init
    Init -> (junction 5179) : [flag_InitEERead]
    (junction 5179) -> Normal.On [order 1] : [ee_EcoMode == ACTIVE &&...
        | (ee_DeluxeG5 == ACTIVE ||...
        |  CD_DELUXE_GEN5_5_FUNCTION != NON_FUNCTIONAL)]
    (junction 5179) -> (junction 5180) [order 2]
    Normal.On -> Normal.Off : [button_EcoMode]
    Normal.Off -> Normal.On : [button_EcoMode]
    (junction 5180) -> Normal.Off
  state Deluxe_G55
    state Off
      en:
      state_EcoMode = INACTIVE;
    state On
      en:
      state_EcoMode = ACTIVE;
    (default) -> Deluxe_G55.Off
    Deluxe_G55.Off -> Deluxe_G55.On : [dlx_G55_EcoMode == ACTIVE]
    Deluxe_G55.On -> Deluxe_G55.Off : [dlx_G55_EcoMode != ACTIVE]
  (default) -> (junction 5201)
  (junction 5201) -> Normal [order 1] : [CD_DELUXE_GEN5_5_FUNCTION == NON_FUNCTIONAL]
  (junction 5201) -> Deluxe_G55 [order 2]
  data: state_EcoMode output uint8; button_EcoMode input boolean; INACTIVE parameter uint8; ACTIVE parameter uint8; ee_EcoMode data_store_memory double; ee_DeluxeG5 data_store_memory double; flag_InitEERead input boolean; CD_DELUXE_GEN5_5_FUNCTION parameter uint8; NON_FUNCTIONAL parameter uint8; dlx_G55_EcoMode input uint8

== stateflow chart MX_Gtwy_Control/PanelInterface/mod_BatteryIcon/BatteryIcon ==
  settings: executeAtInitialization=1
  state ERROR
    en:icon_Battery = ERROR_FLASH;
  state INACTIVE
    en:icon_Battery = INACTIVE;
  state ACTIVE
    en:icon_Battery = ACTIVE;
  (default) -> INACTIVE
  INACTIVE -> ERROR [order 1] : [(state_Alternator != NORMAL)...
      | || (state_VBattery != NORMAL)]
  ERROR -> INACTIVE : [(state_Alternator == NORMAL)...
      | && (state_VBattery == NORMAL)]
  INACTIVE -> ACTIVE [order 2] : [(state_InfoPrimary == DISPLAY_BATTERY_VOLTAGE)]
  ACTIVE -> INACTIVE [order 1] : [(state_InfoPrimary != DISPLAY_BATTERY_VOLTAGE)]
  ACTIVE -> ERROR [order 2] : [(state_Alternator != NORMAL)...
      | || (state_VBattery != NORMAL)]
  data: state_VBattery input uint8; icon_Battery output uint8; ACTIVE parameter uint8; INACTIVE parameter uint8; NORMAL parameter uint8; state_InfoPrimary input uint8; DISPLAY_BATTERY_VOLTAGE parameter uint8; state_Alternator input uint8; ERROR_FLASH parameter uint8

== stateflow chart MX_Gtwy_Control/PanelInterface/mod_ECTIcon/WarningIcon ==
  settings: executeAtInitialization=1
  state INACTIVE
    en:icon_ECT = INACTIVE;
  state ERROR
    en:icon_ECT = ERROR_FLASH;
  state ACTIVE
    en:icon_ECT = ACTIVE;
  (default) -> INACTIVE
  INACTIVE -> ERROR [order 1] : [(state_ECT == EXTREMELY_HIGH)...
      | || (state_ECT == PRE_SHUTDOWN)...
      | || (state_ECT == SHUTDOWN)]
  ERROR -> INACTIVE : [(state_ECT == HIGH)...
      | || (state_ECT == NORMAL)...
      | || (state_ECT == COLD)]
  ACTIVE -> INACTIVE [order 1] : [(state_ECT == NORMAL)...
      | || (state_ECT == COLD)]
  INACTIVE -> ACTIVE [order 2] : [(state_ECT == HIGH)...
      | || (state_ECT == OORH)...
      | || (state_ECT == OORL)]
  ACTIVE -> ERROR [order 2] : [(state_ECT == EXTREMELY_HIGH)...
      | || (state_ECT == PRE_SHUTDOWN)...
      | || (state_ECT == SHUTDOWN)]
  data: state_ECT input uint8; icon_ECT output uint8; ACTIVE parameter uint8; EXTREMELY_HIGH parameter uint8; INACTIVE parameter uint8; NORMAL parameter uint8; OORH parameter uint8; OORL parameter uint8; SHUTDOWN parameter uint8; HIGH parameter uint8; COLD parameter uint8; ERROR_FLASH parameter uint8; PRE_SHUTDOWN parameter uint8

== stateflow chart MX_Gtwy_Control/PanelInterface/mod_EngineIcon/EngineIcon ==
  settings: executeAtInitialization=1
  state SOLID
    state INACTIVE
      en:icon_Engine = INACTIVE;
    state ACTIVE
      en:icon_Engine = ACTIVE;
    (default) -> INACTIVE
    INACTIVE -> ACTIVE : [(state_StarterOutput != NORMAL)...
        | || (state_StarterRelay != NORMAL)...
        | || (state_EOP == LOW)...
        | || (state_EOP == OORL)...
        | || (state_EOP == OORH)...
        | || ((state_ThrottlePrimary != NORMAL) && (state_ThrottlePrimary != NON_FUNCTIONAL))...
        | || ((lamp_Engine & PROTECT_LAMP_STATUS_MASK) == PROTECT_LAMP_STATUS_ACTIVE)...
        | || ((lamp_Engine & AMBER_LAMP_STATUS_MASK) == AMBER_LAMP_STATUS_ACTIVE)...
        | || (state_EngineSpeedDerate == UNRESPONSIVE)...
        | || ((state_FuelTemp != NORMAL) && (state_FuelTemp != NON_FUNCTIONAL))...
        | || (icon_RPM == ERROR_FLASH && ee_DeluxeG5 == ACTIVE)...
        | || (state_GlowPlugOutput != NORMAL && ee_DeluxeG5 == ACTIVE)...
        | || (state_GlowPlugRelay != NORMAL && ee_DeluxeG5 == ACTIVE)...
        | || (state_TurboPrime != NORMAL)]
    ACTIVE -> INACTIVE : [(state_StarterOutput == NORMAL)...
        | && (state_StarterRelay == NORMAL)...
        | && (state_EOP == NORMAL)...
        | && ((state_ThrottlePrimary == NORMAL) || (state_ThrottlePrimary == NON_FUNCTIONAL))...
        | && ((lamp_Engine & PROTECT_LAMP_STATUS_MASK) != PROTECT_LAMP_STATUS_ACTIVE)...
        | && ((lamp_Engine & AMBER_LAMP_STATUS_MASK) != AMBER_LAMP_STATUS_ACTIVE)...
        | && (state_EngineSpeedDerate != UNRESPONSIVE)...
        | && ((state_FuelTemp == NORMAL) || (state_FuelTemp == NON_FUNCTIONAL))...
        | && (icon_RPM != ERROR_FLASH || ee_DeluxeG5 != ACTIVE)...
        | && (state_GlowPlugOutput == NORMAL || ee_DeluxeG5 != ACTIVE)...
        | && (state_GlowPlugRelay == NORMAL || ee_DeluxeG5 != ACTIVE)...
        | && (state_TurboPrime == NORMAL)]
  state ERROR
    en:icon_Engine = ERROR_FLASH;
  (default) -> SOLID
  SOLID -> ERROR : [(state_EOP == EXTREMELY_LOW)...
      | || (state_EOP == SHUTDOWN)...
      | || (error_FuelSec != NORMAL)...
      | || (state_FuelPriOutput != NORMAL)...
      | || (state_FuelPriRelay != NORMAL)...
      | || (state_HPMatch == FAILURE)...
      | || (state_CommECU == NO_COMMUNICATION)...
      | || ((lamp_Engine & RED_STOP_LAMP_STATUS_MASK) == RED_STOP_LAMP_STATUS_ACTIVE)...
      | || ((lamp_Engine & PROTECT_LAMP_FLASH_MASK) ==PROTECT_LAMP_FLASH_ACTIVE)...
      | || (state_EngineSpeedDerate == SHUTDOWN)...
      | || (state_FuelTemp == SHUTDOWN)]
  ERROR -> SOLID : [((state_EOP == NORMAL) || (state_EOP == LOW))...
      | && (error_FuelSec == NORMAL)...
      | && (state_FuelPriOutput == NORMAL)...
      | && (state_FuelPriRelay == NORMAL)...
      | && (state_HPMatch != FAILURE)...
      | && (state_CommECU != NO_COMMUNICATION)...
      | && ((lamp_Engine & RED_STOP_LAMP_STATUS_MASK) != RED_STOP_LAMP_STATUS_ACTIVE)...
      | && ((lamp_Engine & PROTECT_LAMP_FLASH_MASK) != PROTECT_LAMP_FLASH_ACTIVE)...
      | && (state_EngineSpeedDerate != SHUTDOWN)...
      | && (state_FuelTemp != SHUTDOWN)]
  data: icon_Engine output uint8; state_FuelPriOutput input uint8 [0..255]; state_FuelPriRelay input uint8 [0..255]; error_FuelSec input uint8 [0..255]; state_StarterOutput input uint8; state_StarterRelay input uint8; state_EOP input uint8 [0..255]; ACTIVE parameter uint8; EXTREMELY_LOW parameter uint8; INACTIVE parameter uint8; LOW parameter uint8; NORMAL parameter uint8; SHUTDOWN parameter uint8; OORL parameter uint8; OORH parameter uint8; ERROR_FLASH parameter uint8; state_ThrottlePrimary input uint8; state_CommECU input uint8; NO_COMMUNICATION parameter uint8; lamp_Engine input uint16; PROTECT_LAMP_STATUS_MASK parameter uint16; PROTECT_LAMP_STATUS_ACTIVE parameter uint16; AMBER_LAMP_STATUS_ACTIVE parameter uint16; RED_STOP_LAMP_STATUS_MASK parameter uint16; RED_STOP_LAMP_STATUS_ACTIVE parameter uint16; AMBER_LAMP_STATUS_MASK parameter uint16; NON_FUNCTIONAL parameter uint8; UNRESPONSIVE parameter uint8; state_EngineSpeedDerate input uint8; state_HPMatch input uint8 [0..255]; icon_RPM input uint8; ee_DeluxeG5 data_store_memory double; state_GlowPlugOutput input uint8; state_GlowPlugRelay input uint8; FAILURE parameter uint8; state_FuelTemp input uint8; PROTECT_LAMP_FLASH_ACTIVE parameter uint16; PROTECT_LAMP_FLASH_MASK parameter uint16; state_TurboPrime input uint8

== stateflow chart MX_Gtwy_Control/PanelInterface/mod_FuelLevelIcon/FuelLevel ==
  settings: executeAtInitialization=1
  state INACTIVE
    en:icon_Fuel = INACTIVE;
  state ACTIVE
    en:icon_Fuel = ACTIVE;
  (default) -> INACTIVE
  INACTIVE -> ACTIVE : [(state_FuelLevel != NORMAL)]
  ACTIVE -> INACTIVE : [(state_FuelLevel == NORMAL)]
  data: state_FuelLevel input uint8; icon_Fuel output boolean; ACTIVE parameter uint8; INACTIVE parameter uint8; NORMAL parameter uint8

== stateflow chart MX_Gtwy_Control/PanelInterface/mod_GeneralWarningIcon/WarningIcon ==
  settings: executeAtInitialization=1
  state SOLID
    state INACTIVE
      du:icon_General = INACTIVE;
    state ACTIVE
      en:icon_General = ACTIVE;
    (default) -> INACTIVE
    INACTIVE -> ACTIVE : [(state_SwPwrOutput != NORMAL)...
        | || (state_SwPwrRelay != NORMAL)...
        | || (state_PBValveOutput != NORMAL)...
        | || (state_PBValveRelay != NORMAL)...
        | || (state_SecondAux != ACTIVE && state_SecondAux != INACTIVE)...
        | || (state_SensorSupply1 != NORMAL)...
        | || (state_SensorSupply2 != NORMAL)...
        | || (state_ConstantData != NORMAL)...
        | || (state_DeluxeG5 == NO_COMMUNICATION)...
        | || (state_HVACOutput != NORMAL)...
        | || (state_OffsetBase != ACTIVE && state_OffsetBase != INACTIVE)...
        | || (state_OffsetRod != ACTIVE && state_OffsetRod != INACTIVE )...
        | || (state_OffsetPot == OORL)...
        | || (state_OffsetPot == OORH)...
        | || (state_OffsetPot == NOT_IN_NEUTRAL)...
        | || (state_AuxBase != ACTIVE && state_AuxBase != INACTIVE)...
        | || (state_AuxRod != ACTIVE && state_AuxRod != INACTIVE )...
        | || (state_AuxPot == OORL)...
        | || (state_AuxPot == OORH)...
        | || (state_AuxPot == NOT_IN_NEUTRAL)...
        | || (state_LeftPot == OORL)...
        | || (state_LeftPot == OORH)...
        | || (state_LeftPot == NOT_IN_NEUTRAL)...
        | || (state_RightPot == OORL)...
        | || (state_RightPot == OORH)...
        | || (state_RightPot == NOT_IN_NEUTRAL)...
        | || (state_LoadSense != NORMAL)...
        | || (state_HydExchange != NORMAL)...
        | || (state_CommThrottle == NO_COMMUNICATION)...
        | || (state_CommSecondary == NO_COMMUNICATION)...
        | || (state_CommJogShuttle == NO_COMMUNICATION)...
        | || (state_CommStdPanel == NO_COMMUNICATION)...
        | || (state_CommDeluxeG55 == NO_COMMUNICATION)...
        | || (state_CANThrottle  != NORMAL)...
        | || (ee_ThrottleCalState != NORMAL && CD_CAN_THROTTLE_FUNCTION != NON_FUNCTIONAL)...
        | || (icon_Service == ERROR_FLASH && ee_DeluxeG5 == ACTIVE) ]
    ACTIVE -> INACTIVE : [(state_SwPwrOutput == NORMAL)...
        | && (state_SwPwrRelay == NORMAL)...
        | && (state_PBValveOutput == NORMAL)...
        | && (state_PBValveRelay == NORMAL)...
        | && (state_SecondAux == ACTIVE || state_SecondAux == INACTIVE)...
        | && (state_SensorSupply1 == NORMAL)...
        | && (state_SensorSupply2 == NORMAL)...
        | && (state_DeluxeG5 != NO_COMMUNICATION)...
        | && (state_ConstantData == NORMAL)...
        | && (state_HVACOutput == NORMAL)...
        | && (state_OffsetBase == ACTIVE || state_OffsetBase == INACTIVE)...
        | && (state_OffsetRod == ACTIVE || state_OffsetRod == INACTIVE)...
        | && (state_OffsetPot != OORL)...
        | && (state_OffsetPot != OORH)...
        | && (state_OffsetPot != NOT_IN_NEUTRAL)...
        | && (state_AuxBase == ACTIVE || state_AuxBase == INACTIVE)...
        | && (state_AuxRod == ACTIVE || state_AuxRod == INACTIVE)...
        | && (state_AuxPot != OORL)...
        | && (state_AuxPot != OORH)...
        | && (state_AuxPot != NOT_IN_NEUTRAL)...
        | && (state_LeftPot != OORL)...
        | && (state_LeftPot != OORH)...
        | && (state_LeftPot != NOT_IN_NEUTRAL)...
        | && (state_RightPot != OORL)...
        | && (state_RightPot != OORH)...
        | && (state_RightPot != NOT_IN_NEUTRAL)...
        | && (state_LoadSense == NORMAL)...
        | && (state_HydExchange == NORMAL)...
        | && (state_CommThrottle != NO_COMMUNICATION)...
        | && (state_CommSecondary != NO_COMMUNICATION)...
        | && (state_CommJogShuttle != NO_COMMUNICATION)...
        | && (state_CommStdPanel != NO_COMMUNICATION)...
        | && (state_CommDeluxeG55 != NO_COMMUNICATION)...
        | && (state_CANThrottle == NORMAL)...
        | && (ee_ThrottleCalState == NORMAL || CD_CAN_THROTTLE_FUNCTION == NON_FUNCTIONAL)...
        | && (icon_Service != ERROR_FLASH || ee_DeluxeG5 != ACTIVE)]
  state ERROR
    en:icon_General = ERROR_FLASH;
  (default) -> SOLID
  SOLID -> ERROR : [(state_Key == MULTIPLE)...
      | || (state_LoadMoment != NORMAL)...
      | || (state_KeylessPanel == NO_COMMUNICATION)]
  ERROR -> SOLID : [(state_Key != MULTIPLE)...
      | && (state_LoadMoment == NORMAL)...
      | && (state_KeylessPanel  != NO_COMMUNICATION)]
  data: state_SwPwrOutput input uint8; icon_General output uint8; ACTIVE parameter uint8; INACTIVE parameter uint8; NORMAL parameter uint8; state_SwPwrRelay input uint8; state_SensorSupply1 input uint8; state_SensorSupply2 input uint8; state_Key input uint8; MULTIPLE parameter uint8; ERROR_FLASH parameter uint8; NO_COMMUNICATION parameter uint8; state_ConstantData input uint8; state_KeylessPanel input uint8; state_DeluxeG5 input uint8; state_HVACOutput input uint8; state_CommThrottle input uint8; state_HydExchange input uint8; state_LoadMoment input uint8; state_LoadSense input uint8; state_OffsetPot input uint8; state_OffsetBase input uint8; state_OffsetRod input uint8; state_AuxPot input uint8; state_AuxBase input uint8; state_AuxRod input uint8; state_CommSecondary input uint8; state_CommJogShuttle input uint8; NOT_IN_NEUTRAL parameter uint8; OORH parameter uint8; OORL parameter uint8; state_CANThrottle input uint8; ee_ThrottleCalState data_store_memory double; CD_CAN_THROTTLE_FUNCTION parameter uint8; NON_FUNCTIONAL parameter uint8; state_CommStdPanel input uint8; ee_DeluxeG5 data_store_memory double; icon_Service input double; state_PBValveOutput input uint8; state_PBValveRelay input uint8; state_CommDeluxeG55 input uint8; state_SecondAux input uint8; state_RightPot input uint8; state_LeftPot input uint8

== stateflow chart MX_Gtwy_Control/PanelInterface/mod_GlowPlugIcon/GlowPlugIcon ==
  settings: executeAtInitialization=1
  state ERROR
    en:icon_GlowPlug = ERROR_FLASH;
  state INACTIVE
    en:icon_GlowPlug = INACTIVE;
  state ACTIVE
    en:icon_GlowPlug = ACTIVE;
  (default) -> INACTIVE
  INACTIVE -> ERROR [order 1] : [(state_GlowPlugOutput != NORMAL)...
      | || (state_GlowPlugRelay != NORMAL)]
  ERROR -> INACTIVE : [(state_GlowPlugOutput == NORMAL)...
      | && (state_GlowPlugRelay == NORMAL)]
  INACTIVE -> ACTIVE [order 2] : [(dout_GlowPlug == ACTIVE)...
      | || ((ecu_GlowPlug == ACTIVE) && (state_Engine != ENGINE_RUNNING))]
  ACTIVE -> INACTIVE [order 1] : [(dout_GlowPlug == INACTIVE)...
      | && ((ecu_GlowPlug == INACTIVE) || (state_Engine == ENGINE_RUNNING))]
  ACTIVE -> ERROR [order 2] : [(state_GlowPlugOutput != NORMAL)...
      | || (state_GlowPlugRelay != NORMAL)]
  data: dout_GlowPlug input boolean; state_GlowPlugOutput input uint8; state_GlowPlugRelay input uint8; icon_GlowPlug output uint8; ACTIVE parameter uint8; INACTIVE parameter uint8; NORMAL parameter uint8; ERROR_FLASH parameter uint8; ecu_GlowPlug input uint8; state_Engine input uint8; ENGINE_RUNNING parameter uint8

== matlab function MX_Gtwy_Control/PanelInterface/mod_HourMeterIcon/Truth_Table ==
  data: state_InfoPrimary input uint8; icon_Hourmeter output uint8; state_InfoSecondary input uint8
function icon_Hourmeter = fcn(state_InfoPrimary, state_InfoSecondary)

aVarTruthTableCondition_1 = false;
aVarTruthTableCondition_2 = false;
aVarTruthTableCondition_3 = false;
aVarTruthTableCondition_4 = false;
aVarTruthTableCondition_5 = false;
aVarTruthTableCondition_6 = false;


% Hourmeter

aVarTruthTableCondition_1 = logical(state_InfoPrimary == 0);

% Glow plugs

aVarTruthTableCondition_2 = logical(state_InfoSecondary == 1);

% Service Clock

aVarTruthTableCondition_3 = logical(state_InfoPrimary == 4);

% Job Clock 1

aVarTruthTableCondition_4 = logical(state_InfoPrimary == 6);

% Job Clock 2

aVarTruthTableCondition_5 = logical(state_InfoPrimary == 7);

% Service Clock is in alarm

aVarTruthTableCondition_6 = logical(state_InfoSecondary == 11);

if (aVarTruthTableCondition_1)
    aFcnTruthTableAction_1();
elseif (aVarTruthTableCondition_2)
    aFcnTruthTableAction_1();
elseif (aVarTruthTableCondition_3)
    aFcnTruthTableAction_1();
elseif (aVarTruthTableCondition_4)
    aFcnTruthTableAction_1();
elseif (aVarTruthTableCondition_5)
    aFcnTruthTableAction_1();
elseif (aVarTruthTableCondition_6)
    aFcnTruthTableAction_3();
else % Default
    aFcnTruthTableAction_2();
end

function aFcnTruthTableAction_1()

% Turn on icon

icon_Hourmeter = uint8(1);

function aFcnTruthTableAction_2()

% Turn off icon

icon_Hourmeter = uint8(0);

function aFcnTruthTableAction_3()

% Flash Icon

icon_Hourmeter = uint8(2);
